simulink model "sma_corr"  (Simulink 8.0)
settings: Solver=VariableStepDiscrete  SolverMode=SingleTasking  StartTime=0.0  StopTime=1000  RelTol=1e-3
PreLoadFcn:
  | % Set this to 1 if simulating
  | simulating = 0;
  | 
  | % Overall parameters
  | n_comp = 128;
  | 
  | % Simulation
  | if (simulating)
  |     n_tvg_frames = 4;
  |     frames_per_vector = 4*8;
  |     vectors_per_wstep = 2;
  |     total_wsteps = 1; % 2^n
  |     % Laura's params
  |     x_ctr = 12;
  |     x_data_ctr = x_ctr-5;
  |     rx_addr_ctr = x_data_ctr+2;
  |     tx_addr_ctr = x_data_ctr+2;
  | else
  |     n_tvg_frames = 256;
  |     frames_per_vector = 256*8;
  |     vectors_per_wstep = 11;
  |     total_wsteps = 6; % 2^n
  |     % Laura's params
  |     x_ctr = 32;
  |     x_data_ctr = 18;
  |     rx_addr_ctr = x_data_ctr+2;
  |     tx_addr_ctr = x_data_ctr+2;
  | end
  | 
  | sb_states = repmat([ones(1, 256) zeros(1, 256)], 1, 2^(total_wsteps-1));
InitFcn:
  | % Get the XSG object to find the clock source
  | xps_xsg_blks = find_system(gcs,'FollowLinks','on','LookUnderMasks','all', 'SearchDepth',1, 'Tag','xps:xsg');
  | xsg_obj = xps_block(xps_xsg_blks{1}, {});
  | xsg_obj = xps_xsg(xsg_obj);
  | clk_src = get(xsg_obj, 'clk_src');
  | if strcmp(clk_src, 'adc0_clk')
  |     adc_brd = 'ZDOK 0';
  | elseif strcmp(clk_src, 'adc1_clk')
  |     adc_brd = 'ZDOK 1';
  | else
  |     adc_brd = '';
  | end
  | 
  | adc_clk_rate = 0;
  | % Get the appropriate ADC5g object
  | xps_adc5g_blks = find_system(gcs, 'FollowLinks', 'on', 'LookUnderMasks','all', 'SearchDepth',2, 'Tag','xps:adc5g');
  | for n = 1:length(xps_adc5g_blks)
  |     adc5g_blk = xps_adc5g_blks{n};
  |     if strcmp(get_param(adc5g_blk, 'adc_brd'), adc_brd)
  |         adc_clk_rate = 2*str2num(get_param(adc5g_blk, 'adc_clk_rate'));
  |     end
  | end
undefined symbols (not defined in the file or in its folder): Down, sfp, Up, X, xlipgetpartsetting, Z
libraries: xbsIndex_r4 x9231, casper_library_misc x178, dspsigops x32, casper_library_delays x26, xps_library x21
(part 27 of 92)

== system sma_corr/network/data_fmt/data_weave ==
  note: Woven data format looks like this:
      |
      |         t=128-->|       t=0-->|
      | ..., p0c08[0:127], p0c00[0:127] <-- was bits[127:120]
      | ..., p1c08[0:127], p1c00[0:127]  -- was bits[063:056]
      | ..., p0c09[0:127], p0c01[0:127]  -- was bits[119:112]
      | ..., p1c09[0:127], p1c01[0:127]  -- was bits[055:048]
      |
      | ..., p0c10[0:127], p0c02[0:127]  -- was bits[111:104]
      | ..., p1c10[0:127], p1c02[0:127]  -- was bits[047:040]
      | ..., p0c11[0:127], p0c03[0:127]  -- was bits[103:096]
      | ..., p1c11[0:127], p1c03[0:127]  -- was bits[039:032]
      |
      | ..., p0c12[0:127], p0c04[0:127]  -- was bits[095:088]
      | ..., p1c12[0:127], p1c04[0:127]  -- was bits[031:024]
      | ..., p0c13[0:127], p0c05[0:127]  -- was bits[087:080]
      | ..., p1c13[0:127], p1c05[0:127]  -- was bits[023:016]
      |
      | ..., p0c14[0:127], p0c06[0:127]  -- was bits[079:072]
      | ..., p1c14[0:127], p1c06[0:127]  -- was bits[015:008]
      | ..., p0c15[0:127], p0c07[0:127]  -- was bits[071:064]
      | ..., p1c15[0:127], p1c07[0:127] <-- was bits[007:000]
  note: Concatenated data looks like this:
      |
      |         t=128-->|       t=0-->|
      | ..., p0c08[0:127], p0c00[0:127] <-- bits[127:120]
      | ..., p0c09[0:127], p0c01[0:127]  -- bits[119:112]
      | ..., p0c10[0:127], p0c02[0:127]  -- bits[111:104]
      | ..., p0c11[0:127], p0c03[0:127]  -- bits[103:096]
      | ..., p0c12[0:127], p0c04[0:127]  -- bits[095:088]
      | ..., p0c13[0:127], p0c05[0:127]  -- bits[087:080]
      | ..., p0c14[0:127], p0c06[0:127]  -- bits[079:072]
      | ..., p0c15[0:127], p0c07[0:127]  -- bits[071:064]
      | ..., p1c08[0:127], p1c00[0:127]  -- bits[063:056]
      | ..., p1c09[0:127], p1c01[0:127]  -- bits[055:048]
      | ..., p1c10[0:127], p1c02[0:127]  -- bits[047:040]
      | ..., p1c11[0:127], p1c03[0:127]  -- bits[039:032]
      | ..., p1c12[0:127], p1c04[0:127]  -- bits[031:024]
      | ..., p1c13[0:127], p1c05[0:127]  -- bits[023:016]
      | ..., p1c14[0:127], p1c06[0:127]  -- bits[015:008]
      | ..., p1c15[0:127], p1c07[0:127] <-- bits[007:000]
  d : Inport
  data_weave : xbsIndex_r4/BitBasher  bitexpr=|  display_expr=off  sr_1=1  arith_type1=Unsigned  bin_pt1=0  sr_2=2  arith_type2=Unsigned  bin_pt2=0  sr_3=3  arith_type3=Unsigned  bin_pt3=0  sr_4=4  arith_type4=Unsigned  bin_pt4=0  dbl_ovrd=off  block_type=bitbasher
      | <!DOCTYPE HTML PUBLIC "-//W3C//DTD HTML 4.0//EN" "http://www.w3.org/TR/REC-html40/strict.dtd">
      | <html><head><meta name="qrichtext" content="1" /><style type="text/css">
      | p, li { white-space: pre-wrap; }
      | </style></head><body style=" font-family:'Sans'; font-size:10pt; font-weight:400; font-style:normal;">
      | <p style=" margin-top:0px; margin-bottom:0px; margin-left:0px; margin-right:0px; -qt-block-indent:0; text-indent:0px;"><span style=" font-family:'MS Shell Dlg'; font-size:8pt;">d0={d[127:120],d[63:56],d[119:112],d[55:48]}</span></p>
      | <p style=" margin-top:0px; margin-bottom:0px; margin-left:0px; margin-right:0px; -qt-block-indent:0; text-indent:0px;"><span style=" font-family:'MS Shell Dlg'; font-size:8pt;">d1={d[111:104],d[47:40],d[103:96],d[39:32]}</span></p>
      | <p style=" margin-top:0px; margin-bottom:0px; margin-left:0px; margin-right:0px; -qt-block-indent:0; text-indent:0px;"><span style=" font-family:'MS Shell Dlg'; font-size:8pt;">d2={d[95:88],d[31:24],d[87:80],d[23:16]}</span></p>
      | <p style=" margin-top:0px; margin-bottom:0px; margin-left:0px; margin-right:0px; -qt-block-indent:0; text-indent:0px;"><span style=" font-family:'MS Shell Dlg'; font-size:8pt;">d3={d[79:72],d[15:8],d[71:64],d[7:0]}</span></p>
      | <p style="-qt-paragraph-type:empty; margin-top:0px; margin-bottom:0px; margin-left:0px; margin-right:0px; -qt-block-indent:0; text-indent:0px; font-family:'MS Shell Dlg'; font-size:8pt;"></p></body></html>
  d0 : Outport
  d1 : Outport  Port=2
  d2 : Outport  Port=3
  d3 : Outport  Port=4
  wires:
  data_weave/4 -> d3/1
  data_weave/3 -> d2/1
  data_weave/1 -> d0/1
  d/1 -> data_weave/1
  data_weave/2 -> d1/1

== system sma_corr/network/eth/0 ==
  note: Start reading out when slot
      | 2 is first written to.
  note: Data to buffer is 68 bits wide:
      | {sowf, 1'b0, data[63:32], 1'b0, eof, data[31:0]}
  note: Capture SOWF bit only
      | with first data word
  in : Inport
  re : Inport  Port=2
  BitBasher1 : xbsIndex_r4/BitBasher  bitexpr=|  display_expr=off  sr_1=1  arith_type1=Unsigned  bin_pt1=0  sr_2=2  arith_type2=Unsigned  bin_pt2=0  sr_3=3  arith_type3=Unsigned  bin_pt3=0  sr_4=4  arith_type4=Unsigned  bin_pt4=0  dbl_ovrd=off  block_type=bitbasher
      | <!DOCTYPE HTML PUBLIC "-//W3C//DTD HTML 4.0//EN" "http://www.w3.org/TR/REC-html40/strict.dtd">
      | <html><head><meta name="qrichtext" content="1" /><style type="text/css">
      | p, li { white-space: pre-wrap; }
      | </style></head><body style=" font-family:'Sans'; font-size:10pt; font-weight:400; font-style:normal;">
      | <p style=" margin-top:12px; margin-bottom:12px; margin-left:0px; margin-right:0px; -qt-block-indent:0; text-indent:0px;"><span style=" font-family:'MS Shell Dlg'; font-size:8pt;">out={sowf, 1'b0, data[63:32], 1'b0, eof, data[31:0]}</span></p></body></html>
  BitBasher3 : xbsIndex_r4/BitBasher  bitexpr=|  display_expr=off  sr_1=1  arith_type1=Unsigned  bin_pt1=0  sr_2=2  arith_type2=Unsigned  bin_pt2=0  sr_3=3  arith_type3=Unsigned  bin_pt3=0  sr_4=4  arith_type4=Unsigned  bin_pt4=0  dbl_ovrd=off  block_type=bitbasher
      | <!DOCTYPE HTML PUBLIC "-//W3C//DTD HTML 4.0//EN" "http://www.w3.org/TR/REC-html40/strict.dtd">
      | <html><head><meta name="qrichtext" content="1" /><style type="text/css">
      | p, li { white-space: pre-wrap; }
      | </style></head><body style=" font-family:'Sans'; font-size:10pt; font-weight:400; font-style:normal;">
      | <p style=" margin-top:12px; margin-bottom:12px; margin-left:0px; margin-right:0px; -qt-block-indent:0; text-indent:0px;"><span style=" font-family:'MS Shell Dlg'; font-size:8pt;">sowf={h[20]}<br /></span></p></body></html>
  Constant : xbsIndex_r4/Constant  const=18008  gui_display_data_type=Fixed-point  arith_type=Unsigned  n_bits=16  bin_pt=0  preci_type=Single  exp_width=8  frac_width=24  explicit_period=off  period=1  equ=P=C  opselect=C  inp2=PCIN>>17  opr=+  inp1=P  carry=CIN  dbl_ovrd=off  block_type=constant
  Register : xbsIndex_r4/Register  init=0  rst=on  en=on  dbl_ovrd=off  block_type=register
  Terminator{1..4} (4 alike) : Terminator
  bsdata : BusSelector  OutputSignals=dout1,valid1,dst1
  bseof : BusSelector  OutputSignals=eof1
  core : xps_library/ten_Gbe_v2  Tag=xps:tengbe_v2  flavour=sfp+  slot=1  port_r1=0  port_r2_cx4=0  port_r2_sfpp=0  rx_dist_ram=off  large_frames=on  show_param=on  pre_emph_r2=0.74  pre_emph=3  post_emph_r2=0.18  rxeqmix_r2=7  swing_r2=940  swing=800  fab_en=on  fab_mac=hex2dec('0200c0a80a01')  fab_ip=192*(2^24) + 168*(2^16) + 10*(2^8) + 1*(2^0)  fab_udp=18008  fab_gate=1  cpu_rx_en=off  cpu_tx_en=off
  depacketizer : Subsystem
  eth_out : Subsystem
  extra_pkt_cnt : Subsystem  Tag=xps:sw_reg  AncestorBlock=xps_library/software register  mask{numios=1, io_dir=To Processor, io_delay=0, sample_period=1, name1=reg, bitwidth1=32, arith_type1=Unsigned, bin_pt1=0}
      mask prompts: numios "Num I/Os"; io_dir "I/O direction"; io_delay "I/O delay"; sample_period "Sample period"; name1 "Name"; bitwidth1 "Bitwidth"; arith_type1 "Type"; bin_pt1 "Binary pt"
      mask init:
      | swreg_init(gcb);
      mask Callback numios: t = timer('StartDelay', 0.1); t.TimerFcn = {@swreg_cb, gcb}; start(t);
      mask TypeOptions io_dir: To Processor | From Processor
      mask Callback io_dir:
      | a = get_param(gcb, 'MaskEnables');
      | if strcmp(get_param(gcb, 'io_dir'), 'To Processor'),
      | a(4) = {'off'};
      | else
      | a(4) = {'on'};
      | end
      | set_param(gcb, 'MaskEnables', a);
      mask TypeOptions arith_type1: Unsigned | Signed  (2's comp) | Boolean
      mask Callback arith_type1:
      | a = get_param(gcb, 'MaskEnables');
      | if strcmp(get_param(gcb, 'arith_type1'), 'Boolean'),
      | set_param(gcb, 'bitwidth1', '1');
      | set_param(gcb, 'bin_pt1', '0');
      | a(6) = {'off'};
      | a(8) = {'off'};
      | else
      | a(6) = {'on'};
      | a(8) = {'on'};
      | end
      | set_param(gcb, 'MaskEnables', a);
  missing_pkt_cnt : Subsystem  Tag=xps:sw_reg  AncestorBlock=xps_library/software register  mask{numios=1, io_dir=To Processor, io_delay=0, sample_period=1, name1=reg, bitwidth1=32, arith_type1=Unsigned, bin_pt1=0}
      mask prompts: numios "Num I/Os"; io_dir "I/O direction"; io_delay "I/O delay"; sample_period "Sample period"; name1 "Name"; bitwidth1 "Bitwidth"; arith_type1 "Type"; bin_pt1 "Binary pt"
      mask init:
      | swreg_init(gcb);
      mask Callback numios: t = timer('StartDelay', 0.1); t.TimerFcn = {@swreg_cb, gcb}; start(t);
      mask TypeOptions io_dir: To Processor | From Processor
      mask Callback io_dir:
      | a = get_param(gcb, 'MaskEnables');
      | if strcmp(get_param(gcb, 'io_dir'), 'To Processor'),
      | a(4) = {'off'};
      | else
      | a(4) = {'on'};
      | end
      | set_param(gcb, 'MaskEnables', a);
      mask TypeOptions arith_type1: Unsigned | Signed  (2's comp) | Boolean
      mask Callback arith_type1:
      | a = get_param(gcb, 'MaskEnables');
      | if strcmp(get_param(gcb, 'arith_type1'), 'Boolean'),
      | set_param(gcb, 'bitwidth1', '1');
      | set_param(gcb, 'bin_pt1', '0');
      | a(6) = {'off'};
      | a(8) = {'off'};
      | else
      | a(6) = {'on'};
      | a(8) = {'on'};
      | end
      | set_param(gcb, 'MaskEnables', a);
  packet_buffer : Subsystem
  pipeline0 : casper_library_delays/pipeline  latency=3
      settings: AttributesFormatString=Latency = %<latency>
  pipeline1 : casper_library_delays/pipeline  latency=3
      settings: AttributesFormatString=Latency = %<latency>
  re_control : Subsystem
  rx_oflows : Subsystem  Tag=xps:sw_reg  AncestorBlock=xps_library/software register  mask{numios=1, io_dir=To Processor, io_delay=0, sample_period=1, name1=reg, bitwidth1=32, arith_type1=Unsigned, bin_pt1=0}
      mask prompts: numios "Num I/Os"; io_dir "I/O direction"; io_delay "I/O delay"; sample_period "Sample period"; name1 "Name"; bitwidth1 "Bitwidth"; arith_type1 "Type"; bin_pt1 "Binary pt"
      mask init:
      | swreg_init(gcb);
      mask Callback numios: t = timer('StartDelay', 0.1); t.TimerFcn = {@swreg_cb, gcb}; start(t);
      mask TypeOptions io_dir: To Processor | From Processor
      mask Callback io_dir:
      | a = get_param(gcb, 'MaskEnables');
      | if strcmp(get_param(gcb, 'io_dir'), 'To Processor'),
      | a(4) = {'off'};
      | else
      | a(4) = {'on'};
      | end
      | set_param(gcb, 'MaskEnables', a);
      mask TypeOptions arith_type1: Unsigned | Signed  (2's comp) | Boolean
      mask Callback arith_type1:
      | a = get_param(gcb, 'MaskEnables');
      | if strcmp(get_param(gcb, 'arith_type1'), 'Boolean'),
      | set_param(gcb, 'bitwidth1', '1');
      | set_param(gcb, 'bin_pt1', '0');
      | a(6) = {'off'};
      | a(8) = {'off'};
      | else
      | a(6) = {'on'};
      | a(8) = {'on'};
      | end
      | set_param(gcb, 'MaskEnables', a);
  tx_oflows : Subsystem  Tag=xps:sw_reg  AncestorBlock=xps_library/software register  mask{numios=1, io_dir=To Processor, io_delay=0, sample_period=1, name1=reg, bitwidth1=32, arith_type1=Unsigned, bin_pt1=0}
      mask prompts: numios "Num I/Os"; io_dir "I/O direction"; io_delay "I/O delay"; sample_period "Sample period"; name1 "Name"; bitwidth1 "Bitwidth"; arith_type1 "Type"; bin_pt1 "Binary pt"
      mask init:
      | swreg_init(gcb);
      mask Callback numios: t = timer('StartDelay', 0.1); t.TimerFcn = {@swreg_cb, gcb}; start(t);
      mask TypeOptions io_dir: To Processor | From Processor
      mask Callback io_dir:
      | a = get_param(gcb, 'MaskEnables');
      | if strcmp(get_param(gcb, 'io_dir'), 'To Processor'),
      | a(4) = {'off'};
      | else
      | a(4) = {'on'};
      | end
      | set_param(gcb, 'MaskEnables', a);
      mask TypeOptions arith_type1: Unsigned | Signed  (2's comp) | Boolean
      mask Callback arith_type1:
      | a = get_param(gcb, 'MaskEnables');
      | if strcmp(get_param(gcb, 'arith_type1'), 'Boolean'),
      | set_param(gcb, 'bitwidth1', '1');
      | set_param(gcb, 'bin_pt1', '0');
      | a(6) = {'off'};
      | a(8) = {'off'};
      | else
      | a(6) = {'on'};
      | a(8) = {'on'};
      | end
      | set_param(gcb, 'MaskEnables', a);
  write_ctrl : Subsystem
  dout : Outport
  pcnt : Outport  Port=2
  hdr : Outport  Port=3
  re_out : Outport  Port=4
  tag scope: rx_bad_0=global, rx_oflows_0=global, rx_pkts_0=global, rx_size_0=global, src_ip_0=global, src_port_0=global, status_0=global, tx_oflows_0=global
  wires:
  eth_out/2(rx_size) -> [rx_size_0]
  core/7 -> eth_out/7(rx_valid_in)
  eth_out/3(rx_pkts) -> [rx_pkts_0]
  core/10 -> eth_out/10(rx_eof)
  core/2 -> eth_out/2(led_rx)
  core/1 -> eth_out/1(led_up)
  eth_out/1(status) -> [status_0]
  eth_out/6(tx_oflows) -> [tx_oflows_0]
  eth_out/5(rx_oflows) -> [rx_oflows_0]
  eth_out/7(rx_src_ip) -> [src_ip_0]
  core/12 -> eth_out/12(rx_overrun_in)
  eth_out/9(rx_data) -> depacketizer/1(data_in)
  core/4 -> eth_out/4(tx_afull)
  core/5 -> eth_out/5(tx_oflow)
  core/11 -> eth_out/11(rx_bad_frame)
  eth_out/4(rx_bad) -> [rx_bad_0]
  core/9 -> eth_out/9(rx_src_port_in)
  eth_out/12(rx_overrun) -> core/8
  core/6 -> eth_out/6(rx_data_in)
  eth_out/8(rx_src_port) -> [src_port_0]
  core/3 -> eth_out/3(led_tx)
  core/8 -> eth_out/8(rx_src_ip_in)
  eth_out/10(rx_valid) -> core/7, depacketizer/2(valid_in)
  bsdata/2 -> core/3  "<valid1>"
  Constant/1 -> core/5
  bsdata/3 -> core/4  "<dst1>"
  eth_out/11(eof) -> depacketizer/3(eof)
  depacketizer/3(data_out) -> BitBasher1/1
  depacketizer/5(eof_out) -> BitBasher1/2
  BitBasher1/1 -> [rxdata]
  depacketizer/4(data_valid) -> Register/2, write_ctrl/2(dv)
  write_ctrl/1(addr) -> [wr_addr]
  write_ctrl/2(we) -> [we]
  [rxdata] -> packet_buffer/3(din)
  [we] -> packet_buffer/4(we)
  [net_rx_rst] -> packet_buffer/1(rst)
  [net_eth_rst] -> core/1
  re/1 -> packet_buffer/5(re)
  packet_buffer/2(pcnt) -> pcnt/1
  depacketizer/2(hdr_valid) -> Register/3
  packet_buffer/1(dout) -> dout/1
  in/1 -> bseof/1, bsdata/1
  bsdata/1 -> core/2  "<dout1>"
  bseof/1 -> core/6  "<eof1>"
  depacketizer/1(hdr) -> BitBasher3/1, write_ctrl/1(hdr), hdr/1
  BitBasher3/1 -> Register/1
  Register/1 -> BitBasher1/3
  depacketizer/6(data_cnt) -> write_ctrl/3(data_cnt)
  [wr_addr] -> packet_buffer/2(wr_addr)
  [wr_addr] -> re_control/1(wr_addr)
  [we] -> re_control/2(we)
  re_control/1(re_out) -> re_out/1
  missing_pkt_cnt/1(sim_out) -> Terminator1/1
  packet_buffer/3(missing) -> missing_pkt_cnt/1(out_reg)
  extra_pkt_cnt/1(sim_out) -> Terminator2/1
  packet_buffer/4(extra) -> extra_pkt_cnt/1(out_reg)
  rx_oflows/1(sim_out) -> Terminator3/1
  tx_oflows/1(sim_out) -> Terminator4/1
  [rx_oflows_0] -> pipeline0/1
  pipeline0/1 -> rx_oflows/1(out_reg)
  pipeline1/1 -> tx_oflows/1(out_reg)
  [tx_oflows_0] -> pipeline1/1

== system sma_corr/network/eth/1 ==
  note: Capture SOWF bit only
      | with first data word
  note: Data to buffer is 68 bits wide:
      | {sowf, 1'b0, data[63:32], 1'b0, eof, data[31:0]}
  note: Start reading out when slot
      | 2 is first written to.
  in : Inport
  re : Inport  Port=2
  BitBasher1 : xbsIndex_r4/BitBasher  bitexpr=|  display_expr=off  sr_1=1  arith_type1=Unsigned  bin_pt1=0  sr_2=2  arith_type2=Unsigned  bin_pt2=0  sr_3=3  arith_type3=Unsigned  bin_pt3=0  sr_4=4  arith_type4=Unsigned  bin_pt4=0  dbl_ovrd=off  block_type=bitbasher
      | <!DOCTYPE HTML PUBLIC "-//W3C//DTD HTML 4.0//EN" "http://www.w3.org/TR/REC-html40/strict.dtd">
      | <html><head><meta name="qrichtext" content="1" /><style type="text/css">
      | p, li { white-space: pre-wrap; }
      | </style></head><body style=" font-family:'Sans'; font-size:10pt; font-weight:400; font-style:normal;">
      | <p style=" margin-top:12px; margin-bottom:12px; margin-left:0px; margin-right:0px; -qt-block-indent:0; text-indent:0px;"><span style=" font-family:'MS Shell Dlg'; font-size:8pt;">out={sowf, 1'b0, data[63:32], 1'b0, eof, data[31:0]}</span></p></body></html>
  BitBasher3 : xbsIndex_r4/BitBasher  bitexpr=|  display_expr=off  sr_1=1  arith_type1=Unsigned  bin_pt1=0  sr_2=2  arith_type2=Unsigned  bin_pt2=0  sr_3=3  arith_type3=Unsigned  bin_pt3=0  sr_4=4  arith_type4=Unsigned  bin_pt4=0  dbl_ovrd=off  block_type=bitbasher
      | <!DOCTYPE HTML PUBLIC "-//W3C//DTD HTML 4.0//EN" "http://www.w3.org/TR/REC-html40/strict.dtd">
      | <html><head><meta name="qrichtext" content="1" /><style type="text/css">
      | p, li { white-space: pre-wrap; }
      | </style></head><body style=" font-family:'Sans'; font-size:10pt; font-weight:400; font-style:normal;">
      | <p style=" margin-top:12px; margin-bottom:12px; margin-left:0px; margin-right:0px; -qt-block-indent:0; text-indent:0px;"><span style=" font-family:'MS Shell Dlg'; font-size:8pt;">sowf={h[20]}<br /></span></p></body></html>
  Constant : xbsIndex_r4/Constant  const=18008  gui_display_data_type=Fixed-point  arith_type=Unsigned  n_bits=16  bin_pt=0  preci_type=Single  exp_width=8  frac_width=24  explicit_period=off  period=1  equ=P=C  opselect=C  inp2=PCIN>>17  opr=+  inp1=P  carry=CIN  dbl_ovrd=off  block_type=constant
  Register : xbsIndex_r4/Register  init=0  rst=on  en=on  dbl_ovrd=off  block_type=register
  Terminator{1..4} (4 alike) : Terminator
  bsdata : BusSelector  OutputSignals=dout0,valid0,dst0
  bseof : BusSelector  OutputSignals=eof0
  core : xps_library/ten_Gbe_v2  Tag=xps:tengbe_v2  flavour=sfp+  slot=1  port_r1=0  port_r2_cx4=0  port_r2_sfpp=1  rx_dist_ram=off  large_frames=on  show_param=on  pre_emph_r2=0.74  pre_emph=3  post_emph_r2=0.18  rxeqmix_r2=7  swing_r2=940  swing=800  fab_en=on  fab_mac=hex2dec('0200c0a80a01')  fab_ip=192*(2^24) + 168*(2^16) + 10*(2^8) + 1*(2^0)  fab_udp=18008  fab_gate=1  cpu_rx_en=off  cpu_tx_en=off
  depacketizer : Subsystem
  eth_out : Subsystem
  extra_pkt_cnt : Subsystem  Tag=xps:sw_reg  AncestorBlock=xps_library/software register  mask{numios=1, io_dir=To Processor, io_delay=0, sample_period=1, name1=reg, bitwidth1=32, arith_type1=Unsigned, bin_pt1=0}
      mask prompts: numios "Num I/Os"; io_dir "I/O direction"; io_delay "I/O delay"; sample_period "Sample period"; name1 "Name"; bitwidth1 "Bitwidth"; arith_type1 "Type"; bin_pt1 "Binary pt"
      mask init:
      | swreg_init(gcb);
      mask Callback numios: t = timer('StartDelay', 0.1); t.TimerFcn = {@swreg_cb, gcb}; start(t);
      mask TypeOptions io_dir: To Processor | From Processor
      mask Callback io_dir:
      | a = get_param(gcb, 'MaskEnables');
      | if strcmp(get_param(gcb, 'io_dir'), 'To Processor'),
      | a(4) = {'off'};
      | else
      | a(4) = {'on'};
      | end
      | set_param(gcb, 'MaskEnables', a);
      mask TypeOptions arith_type1: Unsigned | Signed  (2's comp) | Boolean
      mask Callback arith_type1:
      | a = get_param(gcb, 'MaskEnables');
      | if strcmp(get_param(gcb, 'arith_type1'), 'Boolean'),
      | set_param(gcb, 'bitwidth1', '1');
      | set_param(gcb, 'bin_pt1', '0');
      | a(6) = {'off'};
      | a(8) = {'off'};
      | else
      | a(6) = {'on'};
      | a(8) = {'on'};
      | end
      | set_param(gcb, 'MaskEnables', a);
  missing_pkt_cnt : Subsystem  Tag=xps:sw_reg  AncestorBlock=xps_library/software register  mask{numios=1, io_dir=To Processor, io_delay=0, sample_period=1, name1=reg, bitwidth1=32, arith_type1=Unsigned, bin_pt1=0}
      mask prompts: numios "Num I/Os"; io_dir "I/O direction"; io_delay "I/O delay"; sample_period "Sample period"; name1 "Name"; bitwidth1 "Bitwidth"; arith_type1 "Type"; bin_pt1 "Binary pt"
      mask init:
      | swreg_init(gcb);
      mask Callback numios: t = timer('StartDelay', 0.1); t.TimerFcn = {@swreg_cb, gcb}; start(t);
      mask TypeOptions io_dir: To Processor | From Processor
      mask Callback io_dir:
      | a = get_param(gcb, 'MaskEnables');
      | if strcmp(get_param(gcb, 'io_dir'), 'To Processor'),
      | a(4) = {'off'};
      | else
      | a(4) = {'on'};
      | end
      | set_param(gcb, 'MaskEnables', a);
      mask TypeOptions arith_type1: Unsigned | Signed  (2's comp) | Boolean
      mask Callback arith_type1:
      | a = get_param(gcb, 'MaskEnables');
      | if strcmp(get_param(gcb, 'arith_type1'), 'Boolean'),
      | set_param(gcb, 'bitwidth1', '1');
      | set_param(gcb, 'bin_pt1', '0');
      | a(6) = {'off'};
      | a(8) = {'off'};
      | else
      | a(6) = {'on'};
      | a(8) = {'on'};
      | end
      | set_param(gcb, 'MaskEnables', a);
  packet_buffer : Subsystem
  pipeline0 : casper_library_delays/pipeline  latency=3
      settings: AttributesFormatString=Latency = %<latency>
  pipeline1 : casper_library_delays/pipeline  latency=3
      settings: AttributesFormatString=Latency = %<latency>
  re_control : Subsystem
  rx_oflows : Subsystem  Tag=xps:sw_reg  AncestorBlock=xps_library/software register  mask{numios=1, io_dir=To Processor, io_delay=0, sample_period=1, name1=reg, bitwidth1=32, arith_type1=Unsigned, bin_pt1=0}
      mask prompts: numios "Num I/Os"; io_dir "I/O direction"; io_delay "I/O delay"; sample_period "Sample period"; name1 "Name"; bitwidth1 "Bitwidth"; arith_type1 "Type"; bin_pt1 "Binary pt"
      mask init:
      | swreg_init(gcb);
      mask Callback numios: t = timer('StartDelay', 0.1); t.TimerFcn = {@swreg_cb, gcb}; start(t);
      mask TypeOptions io_dir: To Processor | From Processor
      mask Callback io_dir:
      | a = get_param(gcb, 'MaskEnables');
      | if strcmp(get_param(gcb, 'io_dir'), 'To Processor'),
      | a(4) = {'off'};
      | else
      | a(4) = {'on'};
      | end
      | set_param(gcb, 'MaskEnables', a);
      mask TypeOptions arith_type1: Unsigned | Signed  (2's comp) | Boolean
      mask Callback arith_type1:
      | a = get_param(gcb, 'MaskEnables');
      | if strcmp(get_param(gcb, 'arith_type1'), 'Boolean'),
      | set_param(gcb, 'bitwidth1', '1');
      | set_param(gcb, 'bin_pt1', '0');
      | a(6) = {'off'};
      | a(8) = {'off'};
      | else
      | a(6) = {'on'};
      | a(8) = {'on'};
      | end
      | set_param(gcb, 'MaskEnables', a);
  tx_oflows : Subsystem  Tag=xps:sw_reg  AncestorBlock=xps_library/software register  mask{numios=1, io_dir=To Processor, io_delay=0, sample_period=1, name1=reg, bitwidth1=32, arith_type1=Unsigned, bin_pt1=0}
      mask prompts: numios "Num I/Os"; io_dir "I/O direction"; io_delay "I/O delay"; sample_period "Sample period"; name1 "Name"; bitwidth1 "Bitwidth"; arith_type1 "Type"; bin_pt1 "Binary pt"
      mask init:
      | swreg_init(gcb);
      mask Callback numios: t = timer('StartDelay', 0.1); t.TimerFcn = {@swreg_cb, gcb}; start(t);
      mask TypeOptions io_dir: To Processor | From Processor
      mask Callback io_dir:
      | a = get_param(gcb, 'MaskEnables');
      | if strcmp(get_param(gcb, 'io_dir'), 'To Processor'),
      | a(4) = {'off'};
      | else
      | a(4) = {'on'};
      | end
      | set_param(gcb, 'MaskEnables', a);
      mask TypeOptions arith_type1: Unsigned | Signed  (2's comp) | Boolean
      mask Callback arith_type1:
      | a = get_param(gcb, 'MaskEnables');
      | if strcmp(get_param(gcb, 'arith_type1'), 'Boolean'),
      | set_param(gcb, 'bitwidth1', '1');
      | set_param(gcb, 'bin_pt1', '0');
      | a(6) = {'off'};
      | a(8) = {'off'};
      | else
      | a(6) = {'on'};
      | a(8) = {'on'};
      | end
      | set_param(gcb, 'MaskEnables', a);
  write_ctrl : Subsystem
  dout : Outport
  pcnt : Outport  Port=2
  hdr : Outport  Port=3
  re_out : Outport  Port=4
  tag scope: rx_bad_1=global, rx_oflows_1=global, rx_pkts_1=global, rx_size_1=global, src_ip_1=global, src_port_1=global, status_1=global, tx_oflows_1=global
  wires:
  packet_buffer/4(extra) -> extra_pkt_cnt/1(out_reg)
  extra_pkt_cnt/1(sim_out) -> Terminator2/1
  packet_buffer/3(missing) -> missing_pkt_cnt/1(out_reg)
  missing_pkt_cnt/1(sim_out) -> Terminator1/1
  re_control/1(re_out) -> re_out/1
  [we] -> re_control/2(we)
  [wr_addr] -> re_control/1(wr_addr)
  [wr_addr] -> packet_buffer/2(wr_addr)
  depacketizer/6(data_cnt) -> write_ctrl/3(data_cnt)
  Register/1 -> BitBasher1/3
  BitBasher3/1 -> Register/1
  depacketizer/1(hdr) -> hdr/1, write_ctrl/1(hdr), BitBasher3/1
  bseof/1 -> core/6  "<eof0>"
  bsdata/1 -> core/2  "<dout0>"
  in/1 -> bsdata/1, bseof/1
  packet_buffer/1(dout) -> dout/1
  depacketizer/2(hdr_valid) -> Register/3
  packet_buffer/2(pcnt) -> pcnt/1
  re/1 -> packet_buffer/5(re)
  [net_eth_rst] -> core/1
  [net_rx_rst] -> packet_buffer/1(rst)
  [we] -> packet_buffer/4(we)
  [rxdata] -> packet_buffer/3(din)
  write_ctrl/2(we) -> [we]
  write_ctrl/1(addr) -> [wr_addr]
  depacketizer/4(data_valid) -> write_ctrl/2(dv), Register/2
  BitBasher1/1 -> [rxdata]
  depacketizer/5(eof_out) -> BitBasher1/2
  depacketizer/3(data_out) -> BitBasher1/1
  eth_out/11(eof) -> depacketizer/3(eof)
  bsdata/3 -> core/4  "<dst0>"
  Constant/1 -> core/5
  bsdata/2 -> core/3  "<valid0>"
  eth_out/10(rx_valid) -> depacketizer/2(valid_in), core/7
  core/8 -> eth_out/8(rx_src_ip_in)
  core/3 -> eth_out/3(led_tx)
  eth_out/8(rx_src_port) -> [src_port_1]
  core/6 -> eth_out/6(rx_data_in)
  eth_out/12(rx_overrun) -> core/8
  core/9 -> eth_out/9(rx_src_port_in)
  eth_out/4(rx_bad) -> [rx_bad_1]
  core/11 -> eth_out/11(rx_bad_frame)
  core/5 -> eth_out/5(tx_oflow)
  core/4 -> eth_out/4(tx_afull)
  eth_out/9(rx_data) -> depacketizer/1(data_in)
  core/12 -> eth_out/12(rx_overrun_in)
  eth_out/7(rx_src_ip) -> [src_ip_1]
  eth_out/5(rx_oflows) -> [rx_oflows_1]
  eth_out/6(tx_oflows) -> [tx_oflows_1]
  eth_out/1(status) -> [status_1]
  core/1 -> eth_out/1(led_up)
  core/2 -> eth_out/2(led_rx)
  core/10 -> eth_out/10(rx_eof)
  eth_out/3(rx_pkts) -> [rx_pkts_1]
  core/7 -> eth_out/7(rx_valid_in)
  eth_out/2(rx_size) -> [rx_size_1]
  rx_oflows/1(sim_out) -> Terminator3/1
  tx_oflows/1(sim_out) -> Terminator4/1
  [rx_oflows_1] -> pipeline0/1
  pipeline0/1 -> rx_oflows/1(out_reg)
  pipeline1/1 -> tx_oflows/1(out_reg)
  [tx_oflows_1] -> pipeline1/1

== system sma_corr/network/eth/2 ==
  note: Start reading out when slot
      | 2 is first written to.
  note: Data to buffer is 68 bits wide:
      | {sowf, 1'b0, data[63:32], 1'b0, eof, data[31:0]}
  note: Capture SOWF bit only
      | with first data word
  in : Inport
  re : Inport  Port=2
  BitBasher1 : xbsIndex_r4/BitBasher  bitexpr=|  display_expr=off  sr_1=1  arith_type1=Unsigned  bin_pt1=0  sr_2=2  arith_type2=Unsigned  bin_pt2=0  sr_3=3  arith_type3=Unsigned  bin_pt3=0  sr_4=4  arith_type4=Unsigned  bin_pt4=0  dbl_ovrd=off  block_type=bitbasher
      | <!DOCTYPE HTML PUBLIC "-//W3C//DTD HTML 4.0//EN" "http://www.w3.org/TR/REC-html40/strict.dtd">
      | <html><head><meta name="qrichtext" content="1" /><style type="text/css">
      | p, li { white-space: pre-wrap; }
      | </style></head><body style=" font-family:'Sans'; font-size:10pt; font-weight:400; font-style:normal;">
      | <p style=" margin-top:12px; margin-bottom:12px; margin-left:0px; margin-right:0px; -qt-block-indent:0; text-indent:0px;"><span style=" font-family:'MS Shell Dlg'; font-size:8pt;">out={sowf, 1'b0, data[63:32], 1'b0, eof, data[31:0]}</span></p></body></html>
  BitBasher3 : xbsIndex_r4/BitBasher  bitexpr=|  display_expr=off  sr_1=1  arith_type1=Unsigned  bin_pt1=0  sr_2=2  arith_type2=Unsigned  bin_pt2=0  sr_3=3  arith_type3=Unsigned  bin_pt3=0  sr_4=4  arith_type4=Unsigned  bin_pt4=0  dbl_ovrd=off  block_type=bitbasher
      | <!DOCTYPE HTML PUBLIC "-//W3C//DTD HTML 4.0//EN" "http://www.w3.org/TR/REC-html40/strict.dtd">
      | <html><head><meta name="qrichtext" content="1" /><style type="text/css">
      | p, li { white-space: pre-wrap; }
      | </style></head><body style=" font-family:'Sans'; font-size:10pt; font-weight:400; font-style:normal;">
      | <p style=" margin-top:12px; margin-bottom:12px; margin-left:0px; margin-right:0px; -qt-block-indent:0; text-indent:0px;"><span style=" font-family:'MS Shell Dlg'; font-size:8pt;">sowf={h[20]}<br /></span></p></body></html>
  Constant : xbsIndex_r4/Constant  const=18008  gui_display_data_type=Fixed-point  arith_type=Unsigned  n_bits=16  bin_pt=0  preci_type=Single  exp_width=8  frac_width=24  explicit_period=off  period=1  equ=P=C  opselect=C  inp2=PCIN>>17  opr=+  inp1=P  carry=CIN  dbl_ovrd=off  block_type=constant
  Register : xbsIndex_r4/Register  init=0  rst=on  en=on  dbl_ovrd=off  block_type=register
  Terminator{1..4} (4 alike) : Terminator
  bsdata : BusSelector  OutputSignals=dout3,valid3,dst3
  bseof : BusSelector  OutputSignals=eof3
  core : xps_library/ten_Gbe_v2  Tag=xps:tengbe_v2  flavour=sfp+  slot=1  port_r1=0  port_r2_cx4=0  port_r2_sfpp=2  rx_dist_ram=off  large_frames=on  show_param=on  pre_emph_r2=0.74  pre_emph=3  post_emph_r2=0.18  rxeqmix_r2=7  swing_r2=940  swing=800  fab_en=on  fab_mac=hex2dec('0200c0a80a01')  fab_ip=192*(2^24) + 168*(2^16) + 10*(2^8) + 1*(2^0)  fab_udp=18008  fab_gate=1  cpu_rx_en=off  cpu_tx_en=off
  depacketizer : Subsystem
  eth_out : Subsystem
  extra_pkt_cnt : Subsystem  Tag=xps:sw_reg  AncestorBlock=xps_library/software register  mask{numios=1, io_dir=To Processor, io_delay=0, sample_period=1, name1=reg, bitwidth1=32, arith_type1=Unsigned, bin_pt1=0}
      mask prompts: numios "Num I/Os"; io_dir "I/O direction"; io_delay "I/O delay"; sample_period "Sample period"; name1 "Name"; bitwidth1 "Bitwidth"; arith_type1 "Type"; bin_pt1 "Binary pt"
      mask init:
      | swreg_init(gcb);
      mask Callback numios: t = timer('StartDelay', 0.1); t.TimerFcn = {@swreg_cb, gcb}; start(t);
      mask TypeOptions io_dir: To Processor | From Processor
      mask Callback io_dir:
      | a = get_param(gcb, 'MaskEnables');
      | if strcmp(get_param(gcb, 'io_dir'), 'To Processor'),
      | a(4) = {'off'};
      | else
      | a(4) = {'on'};
      | end
      | set_param(gcb, 'MaskEnables', a);
      mask TypeOptions arith_type1: Unsigned | Signed  (2's comp) | Boolean
      mask Callback arith_type1:
      | a = get_param(gcb, 'MaskEnables');
      | if strcmp(get_param(gcb, 'arith_type1'), 'Boolean'),
      | set_param(gcb, 'bitwidth1', '1');
      | set_param(gcb, 'bin_pt1', '0');
      | a(6) = {'off'};
      | a(8) = {'off'};
      | else
      | a(6) = {'on'};
      | a(8) = {'on'};
      | end
      | set_param(gcb, 'MaskEnables', a);
  missing_pkt_cnt : Subsystem  Tag=xps:sw_reg  AncestorBlock=xps_library/software register  mask{numios=1, io_dir=To Processor, io_delay=0, sample_period=1, name1=reg, bitwidth1=32, arith_type1=Unsigned, bin_pt1=0}
      mask prompts: numios "Num I/Os"; io_dir "I/O direction"; io_delay "I/O delay"; sample_period "Sample period"; name1 "Name"; bitwidth1 "Bitwidth"; arith_type1 "Type"; bin_pt1 "Binary pt"
      mask init:
      | swreg_init(gcb);
      mask Callback numios: t = timer('StartDelay', 0.1); t.TimerFcn = {@swreg_cb, gcb}; start(t);
      mask TypeOptions io_dir: To Processor | From Processor
      mask Callback io_dir:
      | a = get_param(gcb, 'MaskEnables');
      | if strcmp(get_param(gcb, 'io_dir'), 'To Processor'),
      | a(4) = {'off'};
      | else
      | a(4) = {'on'};
      | end
      | set_param(gcb, 'MaskEnables', a);
      mask TypeOptions arith_type1: Unsigned | Signed  (2's comp) | Boolean
      mask Callback arith_type1:
      | a = get_param(gcb, 'MaskEnables');
      | if strcmp(get_param(gcb, 'arith_type1'), 'Boolean'),
      | set_param(gcb, 'bitwidth1', '1');
      | set_param(gcb, 'bin_pt1', '0');
      | a(6) = {'off'};
      | a(8) = {'off'};
      | else
      | a(6) = {'on'};
      | a(8) = {'on'};
      | end
      | set_param(gcb, 'MaskEnables', a);
  packet_buffer : Subsystem
  pipeline0 : casper_library_delays/pipeline  latency=3
      settings: AttributesFormatString=Latency = %<latency>
  pipeline1 : casper_library_delays/pipeline  latency=3
      settings: AttributesFormatString=Latency = %<latency>
  re_control : Subsystem
  rx_oflows : Subsystem  Tag=xps:sw_reg  AncestorBlock=xps_library/software register  mask{numios=1, io_dir=To Processor, io_delay=0, sample_period=1, name1=reg, bitwidth1=32, arith_type1=Unsigned, bin_pt1=0}
      mask prompts: numios "Num I/Os"; io_dir "I/O direction"; io_delay "I/O delay"; sample_period "Sample period"; name1 "Name"; bitwidth1 "Bitwidth"; arith_type1 "Type"; bin_pt1 "Binary pt"
      mask init:
      | swreg_init(gcb);
      mask Callback numios: t = timer('StartDelay', 0.1); t.TimerFcn = {@swreg_cb, gcb}; start(t);
      mask TypeOptions io_dir: To Processor | From Processor
      mask Callback io_dir:
      | a = get_param(gcb, 'MaskEnables');
      | if strcmp(get_param(gcb, 'io_dir'), 'To Processor'),
      | a(4) = {'off'};
      | else
      | a(4) = {'on'};
      | end
      | set_param(gcb, 'MaskEnables', a);
      mask TypeOptions arith_type1: Unsigned | Signed  (2's comp) | Boolean
      mask Callback arith_type1:
      | a = get_param(gcb, 'MaskEnables');
      | if strcmp(get_param(gcb, 'arith_type1'), 'Boolean'),
      | set_param(gcb, 'bitwidth1', '1');
      | set_param(gcb, 'bin_pt1', '0');
      | a(6) = {'off'};
      | a(8) = {'off'};
      | else
      | a(6) = {'on'};
      | a(8) = {'on'};
      | end
      | set_param(gcb, 'MaskEnables', a);
  tx_oflows : Subsystem  Tag=xps:sw_reg  AncestorBlock=xps_library/software register  mask{numios=1, io_dir=To Processor, io_delay=0, sample_period=1, name1=reg, bitwidth1=32, arith_type1=Unsigned, bin_pt1=0}
      mask prompts: numios "Num I/Os"; io_dir "I/O direction"; io_delay "I/O delay"; sample_period "Sample period"; name1 "Name"; bitwidth1 "Bitwidth"; arith_type1 "Type"; bin_pt1 "Binary pt"
      mask init:
      | swreg_init(gcb);
      mask Callback numios: t = timer('StartDelay', 0.1); t.TimerFcn = {@swreg_cb, gcb}; start(t);
      mask TypeOptions io_dir: To Processor | From Processor
      mask Callback io_dir:
      | a = get_param(gcb, 'MaskEnables');
      | if strcmp(get_param(gcb, 'io_dir'), 'To Processor'),
      | a(4) = {'off'};
      | else
      | a(4) = {'on'};
      | end
      | set_param(gcb, 'MaskEnables', a);
      mask TypeOptions arith_type1: Unsigned | Signed  (2's comp) | Boolean
      mask Callback arith_type1:
      | a = get_param(gcb, 'MaskEnables');
      | if strcmp(get_param(gcb, 'arith_type1'), 'Boolean'),
      | set_param(gcb, 'bitwidth1', '1');
      | set_param(gcb, 'bin_pt1', '0');
      | a(6) = {'off'};
      | a(8) = {'off'};
      | else
      | a(6) = {'on'};
      | a(8) = {'on'};
      | end
      | set_param(gcb, 'MaskEnables', a);
  write_ctrl : Subsystem
  dout : Outport
  pcnt : Outport  Port=2
  hdr : Outport  Port=3
  re_out : Outport  Port=4
  tag scope: rx_bad_2=global, rx_oflows_2=global, rx_pkts_2=global, rx_size_2=global, src_ip_2=global, src_port_2=global, status_2=global, tx_oflows_2=global
  wires:
  eth_out/2(rx_size) -> [rx_size_2]
  core/7 -> eth_out/7(rx_valid_in)
  eth_out/3(rx_pkts) -> [rx_pkts_2]
  core/10 -> eth_out/10(rx_eof)
  core/2 -> eth_out/2(led_rx)
  core/1 -> eth_out/1(led_up)
  eth_out/1(status) -> [status_2]
  eth_out/6(tx_oflows) -> [tx_oflows_2]
  eth_out/5(rx_oflows) -> [rx_oflows_2]
  eth_out/7(rx_src_ip) -> [src_ip_2]
  core/12 -> eth_out/12(rx_overrun_in)
  eth_out/9(rx_data) -> depacketizer/1(data_in)
  core/4 -> eth_out/4(tx_afull)
  core/5 -> eth_out/5(tx_oflow)
  core/11 -> eth_out/11(rx_bad_frame)
  eth_out/4(rx_bad) -> [rx_bad_2]
  core/9 -> eth_out/9(rx_src_port_in)
  eth_out/12(rx_overrun) -> core/8
  core/6 -> eth_out/6(rx_data_in)
  eth_out/8(rx_src_port) -> [src_port_2]
  core/3 -> eth_out/3(led_tx)
  core/8 -> eth_out/8(rx_src_ip_in)
  eth_out/10(rx_valid) -> core/7, depacketizer/2(valid_in)
  bsdata/2 -> core/3  "<valid3>"
  Constant/1 -> core/5
  bsdata/3 -> core/4  "<dst3>"
  eth_out/11(eof) -> depacketizer/3(eof)
  depacketizer/3(data_out) -> BitBasher1/1
  depacketizer/5(eof_out) -> BitBasher1/2
  BitBasher1/1 -> [rxdata]
  depacketizer/4(data_valid) -> Register/2, write_ctrl/2(dv)
  write_ctrl/1(addr) -> [wr_addr]
  write_ctrl/2(we) -> [we]
  [rxdata] -> packet_buffer/3(din)
  [we] -> packet_buffer/4(we)
  [net_rx_rst] -> packet_buffer/1(rst)
  [net_eth_rst] -> core/1
  re/1 -> packet_buffer/5(re)
  packet_buffer/2(pcnt) -> pcnt/1
  depacketizer/2(hdr_valid) -> Register/3
  packet_buffer/1(dout) -> dout/1
  in/1 -> bseof/1, bsdata/1
  bsdata/1 -> core/2  "<dout3>"
  bseof/1 -> core/6  "<eof3>"
  depacketizer/1(hdr) -> BitBasher3/1, write_ctrl/1(hdr), hdr/1
  BitBasher3/1 -> Register/1
  Register/1 -> BitBasher1/3
  depacketizer/6(data_cnt) -> write_ctrl/3(data_cnt)
  [wr_addr] -> packet_buffer/2(wr_addr)
  [wr_addr] -> re_control/1(wr_addr)
  [we] -> re_control/2(we)
  re_control/1(re_out) -> re_out/1
  missing_pkt_cnt/1(sim_out) -> Terminator1/1
  packet_buffer/3(missing) -> missing_pkt_cnt/1(out_reg)
  extra_pkt_cnt/1(sim_out) -> Terminator2/1
  packet_buffer/4(extra) -> extra_pkt_cnt/1(out_reg)
  rx_oflows/1(sim_out) -> Terminator3/1
  tx_oflows/1(sim_out) -> Terminator4/1
  [rx_oflows_2] -> pipeline0/1
  pipeline0/1 -> rx_oflows/1(out_reg)
  pipeline1/1 -> tx_oflows/1(out_reg)
  [tx_oflows_2] -> pipeline1/1
